simulink model "controller_observer_AllAgents_v5"  (Simulink 8.4)
settings: Solver=ode1  StartTime=0.0  StopTime=inf  FixedStep=60*1  RelTol=1e-3
values from neighbouring files (symbols this model uses):
  Cpw = 4180    [readepJSONout.m]
  m_max = 3    [DesignParameters.m]
  mc_MAX = 2    [DesignParameters.m]
  Tamb_additive_Sensor_fault_time = 60*60*24*(Tamb_day_ofthe_add_fault + Tamb_time_ofthe_add_fault)    (= 129600)    [faults_setting.m]
  Tamb_additive_Sensor_fault_value = 10    [faults_setting.m]
  Tamb_mult_Sensor_fault_time = 60*60*24*(Tamb_day_ofthe_mult_fault + Tamb_time_ofthe_mult_fault)    (= 129600)    [faults_setting.m]
  Tamb_mult_Sensor_fault_value = 1.1    [faults_setting.m]
  Ts = 3600/dat.Timestep.Timestep_0x20_1.number_of_timesteps_per_hour    [readepJSONout.m]
  Tst_C_additive_Sensor_fault_time = 60*60*24*(Tst_C_day_ofthe_add_fault + Tst_C_time_ofthe_add_fault)    (= 129600)    [faults_setting.m]
  Tst_C_additive_Sensor_fault_value = 10    [faults_setting.m]
  Tst_C_mult_Sensor_fault_time = 60*60*24*(Tst_C_day_ofthe_mult_fault + Tst_C_time_ofthe_mult_fault)    (= 129600)    [faults_setting.m]
  Tst_C_mult_Sensor_fault_value = 1.1    [faults_setting.m]
  Tst_H_additive_Sensor_fault_time = 60*60*24*(Tst_H_day_ofthe_add_fault + Tst_H_time_ofthe_add_fault)    (= 129600)    [faults_setting.m]
  Tst_H_additive_Sensor_fault_value = 10    [faults_setting.m]
  Tst_H_mult_Sensor_fault_time = 60*60*24*(Tst_H_day_ofthe_mult_fault + Tst_H_time_ofthe_mult_fault)    (= 129600)    [faults_setting.m]
  Tst_H_mult_Sensor_fault_value = 1.1    [faults_setting.m]
undefined symbols (not defined in the file or in its folder): building, COP, Ehp_max, fsa_E, fsa_ET, fsa_N, fsa_NT, fsa_W, fsa_WT, h_E, h_ET, h_N, h_NT, h_W, h_WT, K_NtoNT, K_NTtoN, Ke_E, Ke_ET, Ke_N, Ke_NT, Ke_WT, Km_E, Km_ET, Km_N, Km_NT, Km_W, Km_WT, Kout_N, Kout_NT, Kout_W, Kout_WT, Kst, Ksta, Kstcij, KstRef, Kw_E, Kw_ET, Kw_N, Kw_NT, Kw_W, Kw_WT
libraries: simulink x61
(part 2 of 36)

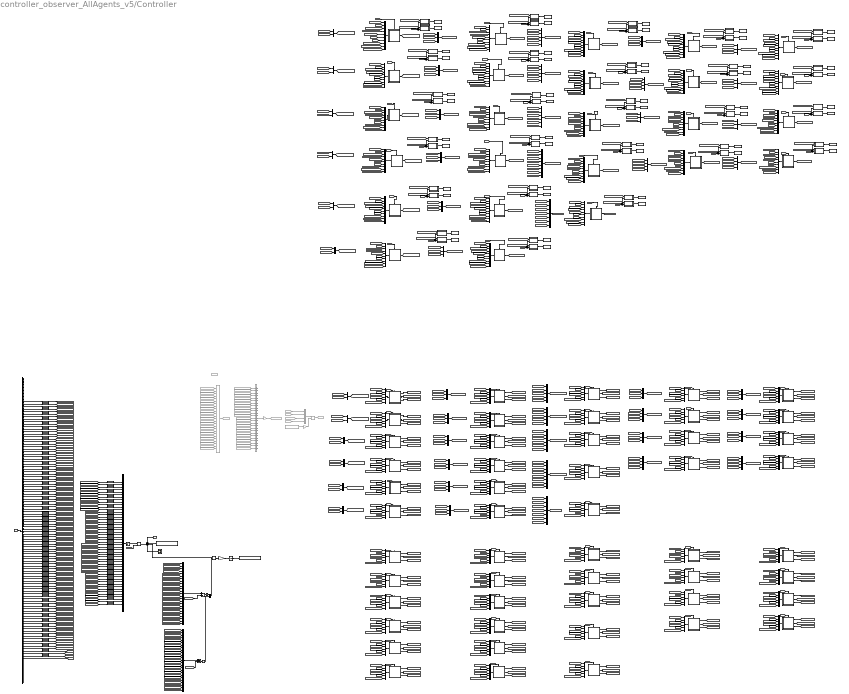
== system controller_observer_AllAgents_v5/Controller ==
  note: Corner Class 1 Pod 1
  note: Inputs/Outputs
  note: Zone ControllersBath_Cooling_
  note: Storage
  note: Corner Class 2 Pod 1
  note: Corner Class 1 Pod 2
  note: Corner Class 2 Pod 2
  note: Corner Class 1 Pod 3
  note: Corner Class 2 Pod 3
  note: Multi Class 1 Pod 1
  note: Multi Class 2 Pod 1
  note: Multi Class 1 Pod 2
  note: Multi Class 2 Pod 2
  note: Multi Class 1 Pod 3
  note: Multi Class 2 Pod 3
  note: Corridor Pod 1
  note: Corridor Pod 2
  note: Corridor Pod 3
  note: Computer Class
  note: Library
  note: Main Corridor
  note: Mech
  note: Offices
  note: Cafeteria
  note: Kitchen
  note: Gym
  note: Lobby
  note: Bath
  note: Storage
  note: Corner Class 1 Pod 1
  note: Zone Controllers
  note: Corner Class 2 Pod 1
  note: Corner Class 1 Pod 2
  note: Corner Class 2 Pod 2
  note: Corner Class 1 Pod 3
  note: Corner Class 2 Pod 3
  note: Multi Class 1 Pod 1
  note: Multi Class 2 Pod 1
  note: Multi Class 1 Pod 2
  note: Multi Class 2 Pod 2
  note: Multi Class 1 Pod 3
  note: Multi Class 2 Pod 3
  note: Corridor Pod 1
  note: Corridor Pod 2
  note: Corridor Pod 3
  note: Computer Class
  note: Library
  note: Main Corridor
  note: Mech
  note: Offices
  note: Cafeteria
  note: Kitchen
  note: Gym
  note: Lobby
  note: Bath
  note: Library
  note: Computer Class
  note: Corridor Pod 1
  note: Corner Class 1 Pod 3
  note: Multi Class 1 Pod 1
  note: Corner Class 1 Pod 1
  note: Corner Class 1 Pod 2
  note: Corner Class 2 Pod 2
  note: Corridor Pod 3
  note: Cafeteria
  note: Multi Class 2 Pod 1
  note: Kitchen
  note: Gym
  note: Lobby
  note: Multi Class 1 Pod 2
  note: Corner Class 2 Pod 3
  note: Multi Class 2 Pod 2
  note: Corridor Pod 2
  note: Diagnosis Agents
  note: Corner Class 2 Pod 1
  note: Main Corridor
  note: Multi Class 2 Pod 3
  note: Offices
  note: Mech
  note: Bath
  note: Multi Class 1 Pod 3
  In1 : Inport
  Abs : Abs
  Abs1 : Abs
  Add : Sum  Commented=on  Inputs=+++++++++++++++++++++++++
  Bath_Agent : Subsystem
  Bath_Cooling : Subsystem
  Bath_Cooling_Mux : Mux  Inputs=[1 1 1 1 1 numel(building.zone(1).neighbour)]
  Bath_Heating : Subsystem
  Bath_Heating_Mux : Mux  Inputs=[1 1 1 1 1 numel(building.zone(1).neighbour)]
  Bath_Mux1 : Mux  Inputs=[1 1 1 1 1 1 1 numel(building.zone(1).neighbour) 1 1]
  Cafeteria_Agent : Subsystem
  Cafeteria_Cooling : Subsystem
  Cafeteria_Cooling_Mux : Mux  Inputs=[1 1 1 1 1 numel(building.zone(2).neighbour)]
  Cafeteria_Heating : Subsystem
  Cafeteria_Heating_Mux : Mux  Inputs=[1 1 1 1 1 numel(building.zone(2).neighbour)]
  Cafeteria_Mux1 : Mux  Inputs=[1 1 1 1 1 1 1 numel(building.zone(2).neighbour) 1 1]
  ComputerClass_Agent : Subsystem
  ComputerClass_Cooling : Subsystem
  ComputerClass_Cooling_Mux : Mux  Inputs=[1 1 1 1 1 numel(building.zone(3).neighbour)]
  ComputerClass_Heating : Subsystem
  ComputerClass_Heating_Mux : Mux  Inputs=[1 1 1 1 1 numel(building.zone(3).neighbour)]
  ComputerClass_Mux1 : Mux  Inputs=[1 1 1 1 1 1 1 numel(building.zone(3).neighbour) 1 1]
  Constant : Constant  Commented=on  Value=[Kst; KstRef; Kstcij; Ksta]
  CornerClass1Pod1_Agent : Subsystem
  CornerClass1Pod1_Cooling : Subsystem
  CornerClass1Pod1_Cooling_Mux : Mux  Inputs=[1 1 1 1 1 numel(building.zone(4).neighbour)]
  CornerClass1Pod1_Heating : Subsystem
  CornerClass1Pod1_Heating_Mux : Mux  Inputs=[1 1 1 1 1 numel(building.zone(4).neighbour)]
  CornerClass1Pod1_Mux1 : Mux  Inputs=[1 1 1 1 1 1 1 numel(building.zone(4).neighbour) 1 1]
  CornerClass1Pod2_Agent : Subsystem
  CornerClass1Pod2_Cooling : Subsystem
  CornerClass1Pod2_Cooling_Mux : Mux  Inputs=[1 1 1 1 1 numel(building.zone(5).neighbour)]
  CornerClass1Pod2_Heating : Subsystem
  CornerClass1Pod2_Heating_Mux : Mux  Inputs=[1 1 1 1 1 numel(building.zone(5).neighbour)]
  CornerClass1Pod2_Mux1 : Mux  Inputs=[1 1 1 1 1 1 1 numel(building.zone(5).neighbour) 1 1]
  CornerClass1Pod3_Agent : Subsystem
  CornerClass1Pod3_Cooling : Subsystem
  CornerClass1Pod3_Cooling_Mux : Mux  Inputs=[1 1 1 1 1 numel(building.zone(6).neighbour)]
  CornerClass1Pod3_Heating : Subsystem
  CornerClass1Pod3_Heating_Mux : Mux  Inputs=[1 1 1 1 1 numel(building.zone(6).neighbour)]
  CornerClass1Pod3_Mux1 : Mux  Inputs=[1 1 1 1 1 1 1 numel(building.zone(6).neighbour) 1 1]
  CornerClass2Pod1_Agent : Subsystem
  CornerClass2Pod1_Cooling : Subsystem
  CornerClass2Pod1_Cooling_Mux : Mux  Inputs=[1 1 1 1 1 numel(building.zone(7).neighbour)]
  CornerClass2Pod1_Heating : Subsystem
  CornerClass2Pod1_Heating_Mux : Mux  Inputs=[1 1 1 1 1 numel(building.zone(7).neighbour)]
  CornerClass2Pod1_Mux1 : Mux  Inputs=[1 1 1 1 1 1 1 numel(building.zone(7).neighbour) 1 1]
  CornerClass2Pod2_Agent : Subsystem
  CornerClass2Pod2_Cooling : Subsystem
  CornerClass2Pod2_Cooling_Mux : Mux  Inputs=[1 1 1 1 1 numel(building.zone(8).neighbour)]
  CornerClass2Pod2_Heating : Subsystem
  CornerClass2Pod2_Heating_Mux : Mux  Inputs=[1 1 1 1 1 numel(building.zone(8).neighbour)]
  CornerClass2Pod2_Mux1 : Mux  Inputs=[1 1 1 1 1 1 1 numel(building.zone(8).neighbour) 1 1]
  CornerClass2Pod3_Agent : Subsystem
  CornerClass2Pod3_Cooling : Subsystem
  CornerClass2Pod3_Cooling_Mux : Mux  Inputs=[1 1 1 1 1 numel(building.zone(9).neighbour)]
  CornerClass2Pod3_Heating : Subsystem
  CornerClass2Pod3_Heating_Mux : Mux  Inputs=[1 1 1 1 1 numel(building.zone(9).neighbour)]
  CornerClass2Pod3_Mux1 : Mux  Inputs=[1 1 1 1 1 1 1 numel(building.zone(9).neighbour) 1 1]
  CorridorPod1_Agent : Subsystem
  CorridorPod1_Cooling : Subsystem
  CorridorPod1_Cooling_Mux : Mux  Inputs=[1 1 1 1 1 numel(building.zone(10).neighbour)]
  CorridorPod1_Heating : Subsystem
  CorridorPod1_Heating_Mux : Mux  Inputs=[1 1 1 1 1 numel(building.zone(10).neighbour)]
  CorridorPod1_Mux1 : Mux  Inputs=[1 1 1 1 1 1 1 numel(building.zone(10).neighbour) 1 1]
  CorridorPod2_Agent : Subsystem
  CorridorPod2_Cooling : Subsystem
  CorridorPod2_Cooling_Mux : Mux  Inputs=[1 1 1 1 1 numel(building.zone(11).neighbour)]
  CorridorPod2_Heating : Subsystem
  CorridorPod2_Heating_Mux : Mux  Inputs=[1 1 1 1 1 numel(building.zone(11).neighbour)]
  CorridorPod2_Mux1 : Mux  Inputs=[1 1 1 1 1 1 1 numel(building.zone(11).neighbour) 1 1]
  CorridorPod3_Agent : Subsystem
  CorridorPod3_Cooling : Subsystem
  CorridorPod3_Cooling_Mux : Mux  Inputs=[1 1 1 1 1 numel(building.zone(12).neighbour)]
  CorridorPod3_Heating : Subsystem
  CorridorPod3_Heating_Mux : Mux  Inputs=[1 1 1 1 1 numel(building.zone(12).neighbour)]
  CorridorPod3_Mux1 : Mux  Inputs=[1 1 1 1 1 1 1 numel(building.zone(12).neighbour) 1 1]
  Cpw : Gain  Gain=Cpw
  Demux : Demux  Outputs=104
  Dot Product : DotProduct  Commented=on  InputSameDT=on  OutDataTypeStr=Inherit: Inherit via internal rule
  EnableD_C_Bath_mat : ToFile  Filename=C:\Users\papad\Dropbox\Complete_building_simulator_Observers\Results_fz4_sensor\EnableD_C_Bath.mat  SaveFormat=Timeseries  SampleTime=Ts
  EnableD_C_Cafeteria_mat : ToFile  Filename=C:\Users\papad\Dropbox\Complete_building_simulator_Observers\Results_fz4_sensor\EnableD_C_Cafeteria.mat  SaveFormat=Timeseries  SampleTime=Ts
  EnableD_C_ComputerClass_mat : ToFile  Filename=C:\Users\papad\Dropbox\Complete_building_simulator_Observers\Results_fz4_sensor\EnableD_C_ComputerClass.mat  SaveFormat=Timeseries  SampleTime=Ts
  EnableD_C_CornerClass1Pod1_mat : ToFile  Filename=C:\Users\papad\Dropbox\Complete_building_simulator_Observers\Results_fz4_sensor\EnableD_C_CornerClass1Pod1.mat  SaveFormat=Timeseries  SampleTime=Ts
  EnableD_C_CornerClass1Pod2_mat : ToFile  Filename=C:\Users\papad\Dropbox\Complete_building_simulator_Observers\Results_fz4_sensor\EnableD_C_CornerClass1Pod2.mat  SaveFormat=Timeseries  SampleTime=Ts
  EnableD_C_CornerClass1Pod3_mat : ToFile  Filename=C:\Users\papad\Dropbox\Complete_building_simulator_Observers\Results_fz4_sensor\EnableD_C_CornerClass1Pod3.mat  SaveFormat=Timeseries  SampleTime=Ts
  EnableD_C_CornerClass2Pod1_mat : ToFile  Filename=C:\Users\papad\Dropbox\Complete_building_simulator_Observers\Results_fz4_sensor\EnableD_C_CornerClass2Pod1.mat  SaveFormat=Timeseries  SampleTime=Ts
  EnableD_C_CornerClass2Pod2_mat : ToFile  Filename=C:\Users\papad\Dropbox\Complete_building_simulator_Observers\Results_fz4_sensor\EnableD_C_CornerClass2Pod2.mat  SaveFormat=Timeseries  SampleTime=Ts
  EnableD_C_CornerClass2Pod3_mat : ToFile  Filename=C:\Users\papad\Dropbox\Complete_building_simulator_Observers\Results_fz4_sensor\EnableD_C_CornerClass2Pod3.mat  SaveFormat=Timeseries  SampleTime=Ts
  EnableD_C_CorridorPod1_mat : ToFile  Filename=C:\Users\papad\Dropbox\Complete_building_simulator_Observers\Results_fz4_sensor\EnableD_C_CorridorPod1.mat  SaveFormat=Timeseries  SampleTime=Ts
  EnableD_C_CorridorPod2_mat : ToFile  Filename=C:\Users\papad\Dropbox\Complete_building_simulator_Observers\Results_fz4_sensor\EnableD_C_CorridorPod2.mat  SaveFormat=Timeseries  SampleTime=Ts
  EnableD_C_CorridorPod3_mat : ToFile  Filename=C:\Users\papad\Dropbox\Complete_building_simulator_Observers\Results_fz4_sensor\EnableD_C_CorridorPod3.mat  SaveFormat=Timeseries  SampleTime=Ts
  EnableD_C_Gym_mat : ToFile  Filename=C:\Users\papad\Dropbox\Complete_building_simulator_Observers\Results_fz4_sensor\EnableD_C_Gym.mat  SaveFormat=Timeseries  SampleTime=Ts
  EnableD_C_Kitchen_mat : ToFile  Filename=C:\Users\papad\Dropbox\Complete_building_simulator_Observers\Results_fz4_sensor\EnableD_C_Kitchen.mat  SaveFormat=Timeseries  SampleTime=Ts
  EnableD_C_LibraryMediaCenter_mat : ToFile  Filename=C:\Users\papad\Dropbox\Complete_building_simulator_Observers\Results_fz4_sensor\EnableD_C_LibraryMediaCenter.mat  SaveFormat=Timeseries  SampleTime=Ts
  EnableD_C_Lobby_mat : ToFile  Filename=C:\Users\papad\Dropbox\Complete_building_simulator_Observers\Results_fz4_sensor\EnableD_C_Lobby.mat  SaveFormat=Timeseries  SampleTime=Ts
  EnableD_C_MainCorridor_mat : ToFile  Filename=C:\Users\papad\Dropbox\Complete_building_simulator_Observers\Results_fz4_sensor\EnableD_C_MainCorridor.mat  SaveFormat=Timeseries  SampleTime=Ts
  EnableD_C_Mech_mat : ToFile  Filename=C:\Users\papad\Dropbox\Complete_building_simulator_Observers\Results_fz4_sensor\EnableD_C_Mech.mat  SaveFormat=Timeseries  SampleTime=Ts
  EnableD_C_MultiClass1Pod1_mat : ToFile  Filename=C:\Users\papad\Dropbox\Complete_building_simulator_Observers\Results_fz4_sensor\EnableD_C_MultiClass1Pod1.mat  SaveFormat=Timeseries  SampleTime=Ts
  EnableD_C_MultiClass1Pod2_mat : ToFile  Filename=C:\Users\papad\Dropbox\Complete_building_simulator_Observers\Results_fz4_sensor\EnableD_C_MultiClass1Pod2.mat  SaveFormat=Timeseries  SampleTime=Ts
  EnableD_C_MultiClass1Pod3_mat : ToFile  Filename=C:\Users\papad\Dropbox\Complete_building_simulator_Observers\Results_fz4_sensor\EnableD_C_MultiClass1Pod3.mat  SaveFormat=Timeseries  SampleTime=Ts
  EnableD_C_MultiClass2Pod1_mat : ToFile  Filename=C:\Users\papad\Dropbox\Complete_building_simulator_Observers\Results_fz4_sensor\EnableD_C_MultiClass2Pod1.mat  SaveFormat=Timeseries  SampleTime=Ts
  EnableD_C_MultiClass2Pod2_mat : ToFile  Filename=C:\Users\papad\Dropbox\Complete_building_simulator_Observers\Results_fz4_sensor\EnableD_C_MultiClass2Pod2.mat  SaveFormat=Timeseries  SampleTime=Ts
  EnableD_C_MultiClass2Pod3_mat : ToFile  Filename=C:\Users\papad\Dropbox\Complete_building_simulator_Observers\Results_fz4_sensor\EnableD_C_MultiClass2Pod3.mat  SaveFormat=Timeseries  SampleTime=Ts
  EnableD_C_Offices_mat : ToFile  Filename=C:\Users\papad\Dropbox\Complete_building_simulator_Observers\Results_fz4_sensor\EnableD_C_Offices.mat  SaveFormat=Timeseries  SampleTime=Ts
  EnableD_H_Bath_mat : ToFile  Filename=C:\Users\papad\Dropbox\Complete_building_simulator_Observers\Results_fz4_sensor\EnableD_H_Bath.mat  SaveFormat=Timeseries  SampleTime=Ts
  EnableD_H_Cafeteria_mat : ToFile  Filename=C:\Users\papad\Dropbox\Complete_building_simulator_Observers\Results_fz4_sensor\EnableD_H_Cafeteria.mat  SaveFormat=Timeseries  SampleTime=Ts
  EnableD_H_ComputerClass_mat : ToFile  Filename=C:\Users\papad\Dropbox\Complete_building_simulator_Observers\Results_fz4_sensor\EnableD_H_ComputerClass.mat  SaveFormat=Timeseries  SampleTime=Ts
  EnableD_H_CornerClass1Pod1_mat : ToFile  Filename=C:\Users\papad\Dropbox\Complete_building_simulator_Observers\Results_fz4_sensor\EnableD_H_CornerClass1Pod1.mat  SaveFormat=Timeseries  SampleTime=Ts
  EnableD_H_CornerClass1Pod2_mat : ToFile  Filename=C:\Users\papad\Dropbox\Complete_building_simulator_Observers\Results_fz4_sensor\EnableD_H_CornerClass1Pod2.mat  SaveFormat=Timeseries  SampleTime=Ts
  EnableD_H_CornerClass1Pod3_mat : ToFile  Filename=C:\Users\papad\Dropbox\Complete_building_simulator_Observers\Results_fz4_sensor\EnableD_H_CornerClass1Pod3.mat  SaveFormat=Timeseries  SampleTime=Ts
  EnableD_H_CornerClass2Pod1_mat : ToFile  Filename=C:\Users\papad\Dropbox\Complete_building_simulator_Observers\Results_fz4_sensor\EnableD_H_CornerClass2Pod1.mat  SaveFormat=Timeseries  SampleTime=Ts
  EnableD_H_CornerClass2Pod2_mat : ToFile  Filename=C:\Users\papad\Dropbox\Complete_building_simulator_Observers\Results_fz4_sensor\EnableD_H_CornerClass2Pod2.mat  SaveFormat=Timeseries  SampleTime=Ts
  EnableD_H_CornerClass2Pod3_mat : ToFile  Filename=C:\Users\papad\Dropbox\Complete_building_simulator_Observers\Results_fz4_sensor\EnableD_H_CornerClass2Pod3.mat  SaveFormat=Timeseries  SampleTime=Ts
  EnableD_H_CorridorPod1_mat : ToFile  Filename=C:\Users\papad\Dropbox\Complete_building_simulator_Observers\Results_fz4_sensor\EnableD_H_CorridorPod1.mat  SaveFormat=Timeseries  SampleTime=Ts
  EnableD_H_CorridorPod2_mat : ToFile  Filename=C:\Users\papad\Dropbox\Complete_building_simulator_Observers\Results_fz4_sensor\EnableD_H_CorridorPod2.mat  SaveFormat=Timeseries  SampleTime=Ts
  EnableD_H_CorridorPod3_mat : ToFile  Filename=C:\Users\papad\Dropbox\Complete_building_simulator_Observers\Results_fz4_sensor\EnableD_H_CorridorPod3.mat  SaveFormat=Timeseries  SampleTime=Ts
  EnableD_H_Gym_mat : ToFile  Filename=C:\Users\papad\Dropbox\Complete_building_simulator_Observers\Results_fz4_sensor\EnableD_H_Gym.mat  SaveFormat=Timeseries  SampleTime=Ts
  EnableD_H_Kitchen_mat : ToFile  Filename=C:\Users\papad\Dropbox\Complete_building_simulator_Observers\Results_fz4_sensor\EnableD_H_Kitchen.mat  SaveFormat=Timeseries  SampleTime=Ts
  EnableD_H_LibraryMediaCenter_mat : ToFile  Filename=C:\Users\papad\Dropbox\Complete_building_simulator_Observers\Results_fz4_sensor\EnableD_H_LibraryMediaCenter.mat  SaveFormat=Timeseries  SampleTime=Ts
  EnableD_H_Lobby_mat : ToFile  Filename=C:\Users\papad\Dropbox\Complete_building_simulator_Observers\Results_fz4_sensor\EnableD_H_Lobby.mat  SaveFormat=Timeseries  SampleTime=Ts
  EnableD_H_MainCorridor_mat : ToFile  Filename=C:\Users\papad\Dropbox\Complete_building_simulator_Observers\Results_fz4_sensor\EnableD_H_MainCorridor.mat  SaveFormat=Timeseries  SampleTime=Ts
  EnableD_H_Mech_mat : ToFile  Filename=C:\Users\papad\Dropbox\Complete_building_simulator_Observers\Results_fz4_sensor\EnableD_H_Mech.mat  SaveFormat=Timeseries  SampleTime=Ts
  EnableD_H_MultiClass1Pod1_mat : ToFile  Filename=C:\Users\papad\Dropbox\Complete_building_simulator_Observers\Results_fz4_sensor\EnableD_H_MultiClass1Pod1.mat  SaveFormat=Timeseries  SampleTime=Ts
  EnableD_H_MultiClass1Pod2_mat : ToFile  Filename=C:\Users\papad\Dropbox\Complete_building_simulator_Observers\Results_fz4_sensor\EnableD_H_MultiClass1Pod2.mat  SaveFormat=Timeseries  SampleTime=Ts
  EnableD_H_MultiClass1Pod3_mat : ToFile  Filename=C:\Users\papad\Dropbox\Complete_building_simulator_Observers\Results_fz4_sensor\EnableD_H_MultiClass1Pod3.mat  SaveFormat=Timeseries  SampleTime=Ts
  EnableD_H_MultiClass2Pod1_mat : ToFile  Filename=C:\Users\papad\Dropbox\Complete_building_simulator_Observers\Results_fz4_sensor\EnableD_H_MultiClass2Pod1.mat  SaveFormat=Timeseries  SampleTime=Ts
  EnableD_H_MultiClass2Pod2_mat : ToFile  Filename=C:\Users\papad\Dropbox\Complete_building_simulator_Observers\Results_fz4_sensor\EnableD_H_MultiClass2Pod2.mat  SaveFormat=Timeseries  SampleTime=Ts
  EnableD_H_MultiClass2Pod3_mat : ToFile  Filename=C:\Users\papad\Dropbox\Complete_building_simulator_Observers\Results_fz4_sensor\EnableD_H_MultiClass2Pod3.mat  SaveFormat=Timeseries  SampleTime=Ts
  EnableD_H_Offices_mat : ToFile  Filename=C:\Users\papad\Dropbox\Complete_building_simulator_Observers\Results_fz4_sensor\EnableD_H_Offices.mat  SaveFormat=Timeseries  SampleTime=Ts
  EnableDifonifmode1 : Subsystem  mask(Enable Diagnosis Agent){}
  EnableDifonifmode10 : Subsystem  mask(Enable Diagnosis Agent){}
  EnableDifonifmode11 : Subsystem  mask(Enable Diagnosis Agent){}
  EnableDifonifmode12 : Subsystem  mask(Enable Diagnosis Agent){}
  EnableDifonifmode13 : Subsystem  mask(Enable Diagnosis Agent){}
  EnableDifonifmode14 : Subsystem  mask(Enable Diagnosis Agent){}
  EnableDifonifmode15 : Subsystem  mask(Enable Diagnosis Agent){}
  EnableDifonifmode16 : Subsystem  mask(Enable Diagnosis Agent){}
  EnableDifonifmode17 : Subsystem  mask(Enable Diagnosis Agent){}
  EnableDifonifmode18 : Subsystem  mask(Enable Diagnosis Agent){}
  EnableDifonifmode19 : Subsystem  mask(Enable Diagnosis Agent){}
  EnableDifonifmode2 : Subsystem  mask(Enable Diagnosis Agent){}
  EnableDifonifmode20 : Subsystem  mask(Enable Diagnosis Agent){}
  EnableDifonifmode21 : Subsystem  mask(Enable Diagnosis Agent){}
  EnableDifonifmode22 : Subsystem  mask(Enable Diagnosis Agent){}
  EnableDifonifmode23 : Subsystem  mask(Enable Diagnosis Agent){}
  EnableDifonifmode24 : Subsystem  mask(Enable Diagnosis Agent){}
  EnableDifonifmode25 : Subsystem  mask(Enable Diagnosis Agent){}
  EnableDifonifmode26 : Subsystem  mask(Enable Diagnosis Agent){}
  EnableDifonifmode27 : Subsystem  mask(Enable Diagnosis Agent){}
  EnableDifonifmode28 : Subsystem  mask(Enable Diagnosis Agent){}
  EnableDifonifmode29 : Subsystem  mask(Enable Diagnosis Agent){}
  EnableDifonifmode3 : Subsystem  mask(Enable Diagnosis Agent){}
  EnableDifonifmode30 : Subsystem  mask(Enable Diagnosis Agent){}
  EnableDifonifmode31 : Subsystem  mask(Enable Diagnosis Agent){}
  EnableDifonifmode32 : Subsystem  mask(Enable Diagnosis Agent){}
  EnableDifonifmode33 : Subsystem  mask(Enable Diagnosis Agent){}
  EnableDifonifmode34 : Subsystem  mask(Enable Diagnosis Agent){}
  EnableDifonifmode35 : Subsystem  mask(Enable Diagnosis Agent){}
  EnableDifonifmode36 : Subsystem  mask(Enable Diagnosis Agent){}
  EnableDifonifmode37 : Subsystem  mask(Enable Diagnosis Agent){}
  EnableDifonifmode38 : Subsystem  mask(Enable Diagnosis Agent){}
  EnableDifonifmode39 : Subsystem  mask(Enable Diagnosis Agent){}
  EnableDifonifmode4 : Subsystem  mask(Enable Diagnosis Agent){}
  EnableDifonifmode40 : Subsystem  mask(Enable Diagnosis Agent){}
  EnableDifonifmode41 : Subsystem  mask(Enable Diagnosis Agent){}
  EnableDifonifmode42 : Subsystem  mask(Enable Diagnosis Agent){}
  EnableDifonifmode43 : Subsystem  mask(Enable Diagnosis Agent){}
  EnableDifonifmode44 : Subsystem  mask(Enable Diagnosis Agent){}
  EnableDifonifmode45 : Subsystem  mask(Enable Diagnosis Agent){}
  EnableDifonifmode46 : Subsystem  mask(Enable Diagnosis Agent){}
  EnableDifonifmode47 : Subsystem  mask(Enable Diagnosis Agent){}
  EnableDifonifmode48 : Subsystem  mask(Enable Diagnosis Agent){}
  EnableDifonifmode49 : Subsystem  mask(Enable Diagnosis Agent){}
  EnableDifonifmode5 : Subsystem  mask(Enable Diagnosis Agent){}
  EnableDifonifmode50 : Subsystem  mask(Enable Diagnosis Agent){}
  EnableDifonifmode6 : Subsystem  mask(Enable Diagnosis Agent){}
  EnableDifonifmode7 : Subsystem  mask(Enable Diagnosis Agent){}
  EnableDifonifmode8 : Subsystem  mask(Enable Diagnosis Agent){}
  EnableDifonifmode9 : Subsystem  mask(Enable Diagnosis Agent){}
  Energy : ToFile  Filename=C:\Users\papad\Dropbox\Complete_building_simulator_Observers\Results_fz4_sensor\EnergyResults_fz4_sensor.mat  SaveFormat=Timeseries
  EnergyIntegrator : DiscreteIntegrator  SampleTime=Ts
  Fault_Tamb : Subsystem
  Fault_Tc_Bath_Cooling : Subsystem
  Fault_Tc_Bath_Heating : Subsystem
  Fault_Tc_Cafeteria_Cooling : Subsystem
  Fault_Tc_Cafeteria_Heating : Subsystem
  Fault_Tc_ComputerClass_Cooling : Subsystem
  Fault_Tc_ComputerClass_Heating : Subsystem
  Fault_Tc_CornerClass1Pod1_Cooling : Subsystem
  Fault_Tc_CornerClass1Pod1_Heating : Subsystem
  Fault_Tc_CornerClass1Pod2_Cooling : Subsystem
  Fault_Tc_CornerClass1Pod2_Heating : Subsystem
  Fault_Tc_CornerClass1Pod3_Cooling : Subsystem
  Fault_Tc_CornerClass1Pod3_Heating : Subsystem
  Fault_Tc_CornerClass2Pod1_Cooling : Subsystem
  Fault_Tc_CornerClass2Pod1_Heating : Subsystem
  Fault_Tc_CornerClass2Pod2_Cooling : Subsystem
  Fault_Tc_CornerClass2Pod2_Heating : Subsystem
  Fault_Tc_CornerClass2Pod3_Cooling : Subsystem
  Fault_Tc_CornerClass2Pod3_Heating : Subsystem
  Fault_Tc_CorridorPod1_Cooling : Subsystem
  Fault_Tc_CorridorPod1_Heating : Subsystem
  Fault_Tc_CorridorPod2_Cooling : Subsystem
  Fault_Tc_CorridorPod2_Heating : Subsystem
  Fault_Tc_CorridorPod3_Cooling : Subsystem
  Fault_Tc_CorridorPod3_Heating : Subsystem
  Fault_Tc_Gym_Cooling : Subsystem
  Fault_Tc_Gym_Heating : Subsystem
  Fault_Tc_Kitchen_Cooling : Subsystem
  Fault_Tc_Kitchen_Heating : Subsystem
  Fault_Tc_LibraryMediaCenter_Cooling : Subsystem
  Fault_Tc_LibraryMediaCenter_Heating : Subsystem
  Fault_Tc_Lobby_Cooling : Subsystem
  Fault_Tc_Lobby_Heating : Subsystem
  Fault_Tc_MainCorridor_Cooling : Subsystem
  Fault_Tc_MainCorridor_Heating : Subsystem
  Fault_Tc_Mech_Cooling : Subsystem
  Fault_Tc_Mech_Heating : Subsystem
  Fault_Tc_MultiClass1Pod1_Cooling : Subsystem
  Fault_Tc_MultiClass1Pod1_Heating : Subsystem
  Fault_Tc_MultiClass1Pod2_Cooling : Subsystem
  Fault_Tc_MultiClass1Pod2_Heating : Subsystem
  Fault_Tc_MultiClass1Pod3_Cooling : Subsystem
  Fault_Tc_MultiClass1Pod3_Heating : Subsystem
  Fault_Tc_MultiClass2Pod1_Cooling : Subsystem
  Fault_Tc_MultiClass2Pod1_Heating : Subsystem
  Fault_Tc_MultiClass2Pod2_Cooling : Subsystem
  Fault_Tc_MultiClass2Pod2_Heating : Subsystem
  Fault_Tc_MultiClass2Pod3_Cooling : Subsystem
  Fault_Tc_MultiClass2Pod3_Heating : Subsystem
  Fault_Tc_Offices_Cooling : Subsystem
  Fault_Tc_Offices_Heating : Subsystem
  Fault_Tsa_Bath : Subsystem
  Fault_Tsa_Cafeteria : Subsystem
  Fault_Tsa_ComputerClass : Subsystem
  Fault_Tsa_CornerClass1Pod1 : Subsystem
  Fault_Tsa_CornerClass1Pod2 : Subsystem
  Fault_Tsa_CornerClass1Pod3 : Subsystem
  Fault_Tsa_CornerClass2Pod1 : Subsystem
  Fault_Tsa_CornerClass2Pod2 : Subsystem
  Fault_Tsa_CornerClass2Pod3 : Subsystem
  Fault_Tsa_CorridorPod1 : Subsystem
  Fault_Tsa_CorridorPod2 : Subsystem
  Fault_Tsa_CorridorPod3 : Subsystem
  Fault_Tsa_Gym : Subsystem
  Fault_Tsa_Kitchen : Subsystem
  Fault_Tsa_LibraryMediaCenter : Subsystem
  Fault_Tsa_Lobby : Subsystem
  Fault_Tsa_MainCorridor : Subsystem
  Fault_Tsa_Mech : Subsystem
  Fault_Tsa_MultiClass1Pod1 : Subsystem
  Fault_Tsa_MultiClass1Pod2 : Subsystem
  Fault_Tsa_MultiClass1Pod3 : Subsystem
  Fault_Tsa_MultiClass2Pod1 : Subsystem
  Fault_Tsa_MultiClass2Pod2 : Subsystem
  Fault_Tsa_MultiClass2Pod3 : Subsystem
  Fault_Tsa_Offices : Subsystem
  Fault_Tst_Cooling : Subsystem
  Fault_Tst_Heating : Subsystem
  Fault_Tz_Bath : Subsystem
  Fault_Tz_Cafeteria : Subsystem
  Fault_Tz_ComputerClass : Subsystem
  Fault_Tz_CornerClass1Pod1 : Subsystem
  Fault_Tz_CornerClass1Pod2 : Subsystem
  Fault_Tz_CornerClass1Pod3 : Subsystem
  Fault_Tz_CornerClass2Pod1 : Subsystem
  Fault_Tz_CornerClass2Pod2 : Subsystem
  Fault_Tz_CornerClass2Pod3 : Subsystem
  Fault_Tz_CorridorPod1 : Subsystem
  Fault_Tz_CorridorPod2 : Subsystem
  Fault_Tz_CorridorPod3 : Subsystem
  Fault_Tz_Gym : Subsystem
  Fault_Tz_Kitchen : Subsystem
  Fault_Tz_LibraryMediaCenter : Subsystem
  Fault_Tz_Lobby : Subsystem
  Fault_Tz_MainCorridor : Subsystem
  Fault_Tz_Mech : Subsystem
  Fault_Tz_MultiClass1Pod1 : Subsystem
  Fault_Tz_MultiClass1Pod2 : Subsystem
  Fault_Tz_MultiClass1Pod3 : Subsystem
  Fault_Tz_MultiClass2Pod1 : Subsystem
  Fault_Tz_MultiClass2Pod2 : Subsystem
  Fault_Tz_MultiClass2Pod3 : Subsystem
  Fault_Tz_Offices : Subsystem
  Fault_mc_Bath_Cooling : Subsystem
  Fault_mc_Bath_Heating : Subsystem
  Fault_mc_Cafeteria_Cooling : Subsystem
  Fault_mc_Cafeteria_Heating : Subsystem
  Fault_mc_ComputerClass_Cooling : Subsystem
  Fault_mc_ComputerClass_Heating : Subsystem
  Fault_mc_CornerClass1Pod1_Cooling : Subsystem
  Fault_mc_CornerClass1Pod1_Heating : Subsystem
  Fault_mc_CornerClass1Pod2_Cooling : Subsystem
  Fault_mc_CornerClass1Pod2_Heating : Subsystem
  Fault_mc_CornerClass1Pod3_Cooling : Subsystem
  Fault_mc_CornerClass1Pod3_Heating : Subsystem
  Fault_mc_CornerClass2Pod1_Cooling : Subsystem
  Fault_mc_CornerClass2Pod1_Heating : Subsystem
  Fault_mc_CornerClass2Pod2_Cooling : Subsystem
  Fault_mc_CornerClass2Pod2_Heating : Subsystem
  Fault_mc_CornerClass2Pod3_Cooling : Subsystem
  Fault_mc_CornerClass2Pod3_Heating : Subsystem
  Fault_mc_CorridorPod1_Cooling : Subsystem
  Fault_mc_CorridorPod1_Heating : Subsystem
  Fault_mc_CorridorPod2_Cooling : Subsystem
  Fault_mc_CorridorPod2_Heating : Subsystem
  Fault_mc_CorridorPod3_Cooling : Subsystem
  Fault_mc_CorridorPod3_Heating : Subsystem
  Fault_mc_Gym_Cooling : Subsystem
  Fault_mc_Gym_Heating : Subsystem
  Fault_mc_Kitchen_Cooling : Subsystem
  Fault_mc_Kitchen_Heating : Subsystem
  Fault_mc_LibraryMediaCenter_Cooling : Subsystem
  Fault_mc_LibraryMediaCenter_Heating : Subsystem
  Fault_mc_Lobby_Cooling : Subsystem
  Fault_mc_Lobby_Heating : Subsystem
  Fault_mc_MainCorridor_Cooling : Subsystem
  Fault_mc_MainCorridor_Heating : Subsystem
  Fault_mc_Mech_Cooling : Subsystem
  Fault_mc_Mech_Heating : Subsystem
  Fault_mc_MultiClass1Pod1_Cooling : Subsystem
  Fault_mc_MultiClass1Pod1_Heating : Subsystem
  Fault_mc_MultiClass1Pod2_Cooling : Subsystem
  Fault_mc_MultiClass1Pod2_Heating : Subsystem
  Fault_mc_MultiClass1Pod3_Cooling : Subsystem
  Fault_mc_MultiClass1Pod3_Heating : Subsystem
  Fault_mc_MultiClass2Pod1_Cooling : Subsystem
  Fault_mc_MultiClass2Pod1_Heating : Subsystem
  Fault_mc_MultiClass2Pod2_Cooling : Subsystem
  Fault_mc_MultiClass2Pod2_Heating : Subsystem
  Fault_mc_MultiClass2Pod3_Cooling : Subsystem
  Fault_mc_MultiClass2Pod3_Heating : Subsystem
  Fault_mc_Offices_Cooling : Subsystem
  Fault_mc_Offices_Heating : Subsystem
  From : From  Commented=on  GotoTag=Ehp
  From10 : From  Commented=on  GotoTag=TstRefCornerClass1Pod1
  From250 : From  Commented=on  GotoTag=TstRefCornerClass2Pod1
  From251 : From  Commented=on  GotoTag=TstRefCornerClass2Pod2
  From252 : From  Commented=on  GotoTag=TstRefCornerClass1Pod2
  From253 : From  Commented=on  GotoTag=TstRefCornerClass2Pod3
  From254 : From  Commented=on  GotoTag=TstRefCornerClass1Pod3
  From255 : From  Commented=on  GotoTag=TstRefMultiClass1Pod1
  From256 : From  Commented=on  GotoTag=TstRefMultiClass2Pod1
  From257 : From  Commented=on  GotoTag=TstRefMultiClass2Pod2
  From258 : From  Commented=on  GotoTag=TstRefMultiClass1Pod2
  From259 : From  Commented=on  GotoTag=TstRefMultiClass2Pod3
  From260 : From  Commented=on  GotoTag=TstRefMultiClass1Pod3
  From261 : From  Commented=on  GotoTag=TstRefCorridorPod1
  From262 : From  Commented=on  GotoTag=TstRefCorridorPod3
  From263 : From  Commented=on  GotoTag=TstRefCorridorPod2
  From264 : From  Commented=on  GotoTag=TstRefMainCorridor
  From265 : From  Commented=on  GotoTag=TstRefMech
  From266 : From  Commented=on  GotoTag=TstRefComputerClass
  From267 : From  Commented=on  GotoTag=TstRefOffices
  From268 : From  Commented=on  GotoTag=TstRefLibrary
  From269 : From  Commented=on  GotoTag=TstRefLobby
  From270 : From  Commented=on  GotoTag=TstRefCafeteria
  From271 : From  Commented=on  GotoTag=TstRefGym
  From272 : From  Commented=on  GotoTag=TstRefKitchen
  From273 : From  Commented=on  GotoTag=TstRefBath
  From274 : From  Commented=on  GotoTag=Mc_Tst_Tc_CornerClass1Pod1
  From275 : From  Commented=on  GotoTag=Mc_Tst_Tc_CornerClass2Pod1
  From276 : From  Commented=on  GotoTag=Mc_Tst_Tc_CornerClass2Pod2
  From277 : From  Commented=on  GotoTag=Mc_Tst_Tc_CornerClass1Pod2
  From278 : From  Commented=on  GotoTag=Mc_Tst_Tc_CornerClass2Pod3
  From279 : From  Commented=on  GotoTag=Mc_Tst_Tc_CornerClass1Pod3
  From280 : From  Commented=on  GotoTag=Mc_Tst_Tc_MultiClass1Pod1
  From281 : From  Commented=on  GotoTag=Mc_Tst_Tc_MultiClass2Pod1
  From282 : From  Commented=on  GotoTag=Mc_Tst_Tc_MultiClass2Pod2
  From283 : From  Commented=on  GotoTag=Mc_Tst_Tc_MultiClass1Pod2
  From284 : From  Commented=on  GotoTag=Mc_Tst_Tc_MultiClass2Pod3
  From285 : From  Commented=on  GotoTag=Mc_Tst_Tc_MultiClass1Pod3
  From286 : From  Commented=on  GotoTag=Mc_Tst_Tc_CorridorPod1
  From287 : From  Commented=on  GotoTag=Mc_Tst_Tc_CorridorPod3
  From288 : From  Commented=on  GotoTag=Mc_Tst_Tc_CorridorPod2
  From289 : From  Commented=on  GotoTag=Mc_Tst_Tc_MainCorridor
  From290 : From  Commented=on  GotoTag=Mc_Tst_Tc_Mech
  From291 : From  Commented=on  GotoTag=Mc_Tst_Tc_ComputerClass
  From292 : From  Commented=on  GotoTag=Mc_Tst_Tc_Offices
  From293 : From  Commented=on  GotoTag=Mc_Tst_Tc_Library
  From294 : From  Commented=on  GotoTag=Mc_Tst_Tc_Lobby
  From295 : From  Commented=on  GotoTag=Mc_Tst_Tc_Cafeteria
  From296 : From  Commented=on  GotoTag=Mc_Tst_Tc_Gym
  From297 : From  Commented=on  GotoTag=Mc_Tst_Tc_Kitchen
  From298 : From  Commented=on  GotoTag=Mc_Tst_Tc_Bath
  From6 : From  Commented=on  GotoTag=Tst_Cooling
  From7 : From  Commented=on  GotoTag=TstRef
  From8 : From  Commented=on  GotoTag=Sum_mc_Tst_Tc
  From9 : From  Commented=on  GotoTag=Tamb
  Gain : Gain  Commented=on  Gain=-1
  Gain1 : Gain  Commented=on  Gain=Cpw/(Ehp_max*COP)
  Goto1 : Goto  Commented=on  GotoTag=Ehp
  Goto2 : Goto  Commented=on  GotoTag=TstRef
  Goto4 : Goto  Commented=on  GotoTag=Sum_mc_Tst_Tc
  Gym_Agent : Subsystem
  Gym_Cooling : Subsystem
  Gym_Cooling_Mux : Mux  Inputs=[1 1 1 1 1 numel(building.zone(13).neighbour)]
  Gym_Heating : Subsystem
  Gym_Heating_Mux : Mux  Inputs=[1 1 1 1 1 numel(building.zone(13).neighbour)]
  Gym_Mux1 : Mux  Inputs=[1 1 1 1 1 1 1 numel(building.zone(13).neighbour) 1 1]
  Kitchen_Agent : Subsystem
  Kitchen_Cooling : Subsystem
  Kitchen_Cooling_Mux : Mux  Inputs=[1 1 1 1 1 numel(building.zone(14).neighbour)]
  Kitchen_Heating : Subsystem
  Kitchen_Heating_Mux : Mux  Inputs=[1 1 1 1 1 numel(building.zone(14).neighbour)]
  Kitchen_Mux1 : Mux  Inputs=[1 1 1 1 1 1 1 numel(building.zone(14).neighbour) 1 1]
  LibraryMediaCenter_Agent : Subsystem
  LibraryMediaCenter_Cooling : Subsystem
  LibraryMediaCenter_Cooling_Mux : Mux  Inputs=[1 1 1 1 1 numel(building.zone(15).neighbour)]
  LibraryMediaCenter_Heating : Subsystem
  LibraryMediaCenter_Heating_Mux : Mux  Inputs=[1 1 1 1 1 numel(building.zone(15).neighbour)]
  LibraryMediaCenter_Mux1 : Mux  Inputs=[1 1 1 1 1 1 1 numel(building.zone(15).neighbour) 1 1]
  Lobby_Agent : Subsystem
  Lobby_Cooling : Subsystem
  Lobby_Cooling_Mux : Mux  Inputs=[1 1 1 1 1 numel(building.zone(16).neighbour)]
  Lobby_Heating : Subsystem
  Lobby_Heating_Mux : Mux  Inputs=[1 1 1 1 1 numel(building.zone(16).neighbour)]
  Lobby_Mux1 : Mux  Inputs=[1 1 1 1 1 1 1 numel(building.zone(16).neighbour) 1 1]
  MainCorridor_Agent : Subsystem
  MainCorridor_Cooling : Subsystem
  MainCorridor_Cooling_Mux : Mux  Inputs=[1 1 1 1 1 numel(building.zone(17).neighbour)]
  MainCorridor_Heating : Subsystem
  MainCorridor_Heating_Mux : Mux  Inputs=[1 1 1 1 1 numel(building.zone(17).neighbour)]
  MainCorridor_Mux1 : Mux  Inputs=[1 1 1 1 1 1 1 numel(building.zone(17).neighbour) 1 1]
  Mech_Agent : Subsystem
  Mech_Cooling : Subsystem
  Mech_Cooling_Mux : Mux  Inputs=[1 1 1 1 1 numel(building.zone(18).neighbour)]
  Mech_Heating : Subsystem
  Mech_Heating_Mux : Mux  Inputs=[1 1 1 1 1 numel(building.zone(18).neighbour)]
  Mech_Mux1 : Mux  Inputs=[1 1 1 1 1 1 1 numel(building.zone(18).neighbour) 1 1]
  MinMax : MinMax  Commented=on  Inputs=25
  MultiClass1Pod1_Agent : Subsystem
  MultiClass1Pod1_Cooling : Subsystem
  MultiClass1Pod1_Cooling_Mux : Mux  Inputs=[1 1 1 1 1 numel(building.zone(19).neighbour)]
  MultiClass1Pod1_Heating : Subsystem
  MultiClass1Pod1_Heating_Mux : Mux  Inputs=[1 1 1 1 1 numel(building.zone(19).neighbour)]
  MultiClass1Pod1_Mux1 : Mux  Inputs=[1 1 1 1 1 1 1 numel(building.zone(19).neighbour) 1 1]
  MultiClass1Pod2_Agent : Subsystem
  MultiClass1Pod2_Cooling : Subsystem
  MultiClass1Pod2_Cooling_Mux : Mux  Inputs=[1 1 1 1 1 numel(building.zone(20).neighbour)]
  MultiClass1Pod2_Heating : Subsystem
  MultiClass1Pod2_Heating_Mux : Mux  Inputs=[1 1 1 1 1 numel(building.zone(20).neighbour)]
  MultiClass1Pod2_Mux1 : Mux  Inputs=[1 1 1 1 1 1 1 numel(building.zone(20).neighbour) 1 1]
  MultiClass1Pod3_Agent : Subsystem
  MultiClass1Pod3_Cooling : Subsystem
  MultiClass1Pod3_Cooling_Mux : Mux  Inputs=[1 1 1 1 1 numel(building.zone(21).neighbour)]
  MultiClass1Pod3_Heating : Subsystem
  MultiClass1Pod3_Heating_Mux : Mux  Inputs=[1 1 1 1 1 numel(building.zone(21).neighbour)]
  MultiClass1Pod3_Mux1 : Mux  Inputs=[1 1 1 1 1 1 1 numel(building.zone(21).neighbour) 1 1]
  MultiClass2Pod1_Agent : Subsystem
  MultiClass2Pod1_Cooling : Subsystem
  MultiClass2Pod1_Cooling_Mux : Mux  Inputs=[1 1 1 1 1 numel(building.zone(22).neighbour)]
  MultiClass2Pod1_Heating : Subsystem
  MultiClass2Pod1_Heating_Mux : Mux  Inputs=[1 1 1 1 1 numel(building.zone(22).neighbour)]
  MultiClass2Pod1_Mux1 : Mux  Inputs=[1 1 1 1 1 1 1 numel(building.zone(22).neighbour) 1 1]
  MultiClass2Pod2_Agent : Subsystem
  MultiClass2Pod2_Cooling : Subsystem
  MultiClass2Pod2_Cooling_Mux : Mux  Inputs=[1 1 1 1 1 numel(building.zone(23).neighbour)]
  MultiClass2Pod2_Heating : Subsystem
  MultiClass2Pod2_Heating_Mux : Mux  Inputs=[1 1 1 1 1 numel(building.zone(23).neighbour)]
  MultiClass2Pod2_Mux1 : Mux  Inputs=[1 1 1 1 1 1 1 numel(building.zone(23).neighbour) 1 1]
  MultiClass2Pod3_Agent : Subsystem
  MultiClass2Pod3_Cooling : Subsystem
  MultiClass2Pod3_Cooling_Mux : Mux  Inputs=[1 1 1 1 1 numel(building.zone(24).neighbour)]
  MultiClass2Pod3_Heating : Subsystem
  MultiClass2Pod3_Heating_Mux : Mux  Inputs=[1 1 1 1 1 numel(building.zone(24).neighbour)]
  MultiClass2Pod3_Mux1 : Mux  Inputs=[1 1 1 1 1 1 1 numel(building.zone(24).neighbour) 1 1]
  Mux : Mux  Inputs=25
  Mux1 : Mux  Commented=on
  Mux{9..14,20,28,30..32,34..39,45,47,49..51} (22 alike) : Mux  Inputs=3
  Mux{15..16,40..41} (4 alike) : Mux  Inputs=5
  Mux17 : Mux  Inputs=6
  Mux18 : Mux  Inputs=8
  Mux19 : Mux  Inputs=7
  Mux2 : Mux  Inputs=50
  Mux{21,29,33,46,48,52} (6 alike) : Mux
  Mux{3..8,22..27,54} (13 alike) : Mux  Inputs=2
  Mux42 : Mux  Inputs=6
  Mux43 : Mux  Inputs=8
  Mux44 : Mux  Inputs=7
  Mux53 : Mux  Inputs=25
  Offices_Agent : Subsystem
  Offices_Cooling : Subsystem
  Offices_Cooling_Mux : Mux  Inputs=[1 1 1 1 1 numel(building.zone(25).neighbour)]
  Offices_Heating : Subsystem
  Offices_Heating_Mux : Mux  Inputs=[1 1 1 1 1 numel(building.zone(25).neighbour)]
  Offices_Mux1 : Mux  Inputs=[1 1 1 1 1 1 1 numel(building.zone(25).neighbour) 1 1]
  Product : Product
  Product1 : Product
  Saturation : Saturate  UpperLimit=mc_MAX  LowerLimit=0  LinearizeAsGain=off  SampleTime=Ts
  Scope : Scope
  Subtract : Sum  Inputs=+-
  Subtract1 : Sum  Inputs=+-
  control_inputs_mat : ToFile  Filename=C:\Users\papad\Dropbox\Complete_building_simulator_Observers\Results_fz4_sensor\control_inputs.mat  SaveFormat=Timeseries  SampleTime=Ts
  Out1 : Outport
  wires:
  Constant/1 -> Gain/1
  Gain/1 -> Dot Product/2
  From6/1 -> Mux1/1
  From7/1 -> Mux1/2
  From8/1 -> Mux1/3
  From9/1 -> Mux1/4
  Mux1/1 -> Dot Product/1
  Dot Product/1 -> Goto1/1
  From10/1 -> MinMax/1
  MinMax/1 -> Goto2/1
  Add/1 -> Gain1/1
  In1/1 -> Demux/1
  [TzCornerClass1Pod1] -> CornerClass1Pod1_Cooling_Mux/1
  [Tst_Cooling] -> CornerClass1Pod1_Cooling_Mux/5
  [Tamb] -> CornerClass1Pod1_Cooling_Mux/2
  CornerClass1Pod1_Cooling/1(Out1) -> [mcCornerClass1Pod1_Cooling]
  [TzNeighborCornerClass1Pod1] -> CornerClass1Pod1_Cooling_Mux/6
  CornerClass1Pod1_Cooling/3(Out3) -> [TstRefCornerClass1Pod1_Cooling]
  [TcCornerClass1Pod1_Cooling] -> CornerClass1Pod1_Cooling_Mux/3
  CornerClass1Pod1_Cooling_Mux/1 -> CornerClass1Pod1_Cooling/1(In1)
  [TsaCornerClass1Pod1] -> CornerClass1Pod1_Cooling_Mux/4
  CornerClass1Pod1_Cooling/2(Out2) -> [Mc_Tst_Tc_CornerClass1Pod1_Cooling]